simulink model "chan_550_clean"  (Simulink 7.3)
settings: Solver=ode45  StartTime=0.0  StopTime=10000  RelTol=1e-3
undefined symbols (not defined in the file): pfb_coeff_gen_calc
libraries: xbsIndex_r4 x3811, casper_library_misc x151, xps_library x50, casper_library_delays x46, casper_library_flow_control x33, casper_library_reorder x4, casper_library_ffts_twiddle x2
(part 2 of 40)

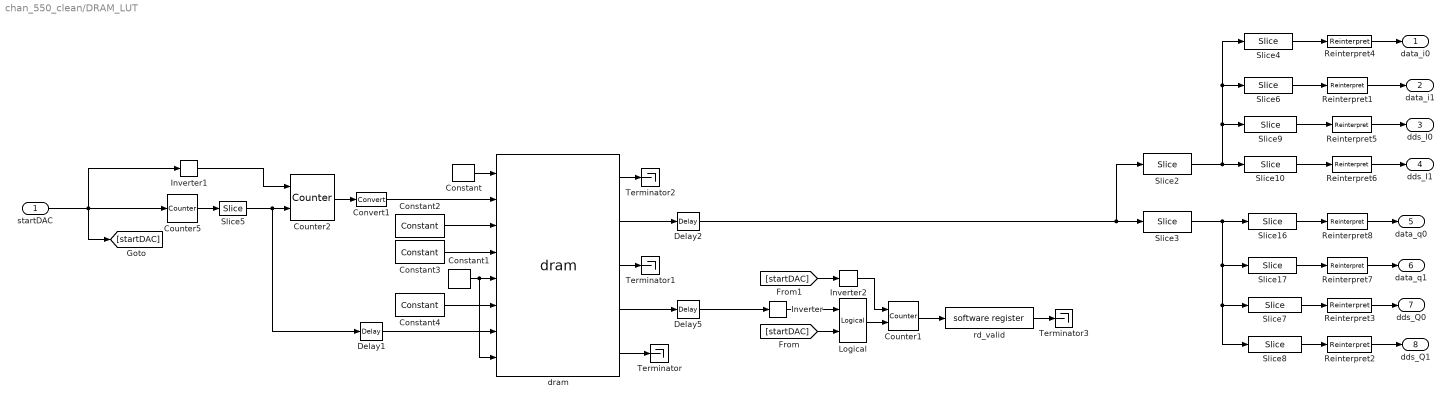
== system chan_550_clean/DRAM_LUT ==
  startDAC : Inport  OutDataType=sfix(16)  OutScaling=2^0
  Constant : xbsIndex_r4/Constant  arith_type=Boolean  const=0  n_bits=16  bin_pt=14  explicit_period=on  period=1  equ=P=C  opselect=C  inp2=PCIN>>17  opr=+  inp1=P  carry=CIN  dbl_ovrd=off  block_type=constant
  Constant1 : xbsIndex_r4/Constant  arith_type=Boolean  const=1  n_bits=16  bin_pt=14  explicit_period=on  period=1  equ=P=C  opselect=C  inp2=PCIN>>17  opr=+  inp1=P  carry=CIN  dbl_ovrd=off  block_type=constant
  Constant2 : xbsIndex_r4/Constant  arith_type=Unsigned  const=0  n_bits=144  bin_pt=0  explicit_period=on  period=1  equ=P=C  opselect=C  inp2=PCIN>>17  opr=+  inp1=P  carry=CIN  dbl_ovrd=off  block_type=constant
  Constant3 : xbsIndex_r4/Constant  arith_type=Unsigned  const=0  n_bits=36  bin_pt=0  explicit_period=on  period=1  equ=P=C  opselect=C  inp2=PCIN>>17  opr=+  inp1=P  carry=CIN  dbl_ovrd=off  block_type=constant
  Constant4 : xbsIndex_r4/Constant  arith_type=Unsigned  const=0  n_bits=32  bin_pt=0  explicit_period=on  period=1  equ=P=C  opselect=C  inp2=PCIN>>17  opr=+  inp1=P  carry=CIN  dbl_ovrd=off  block_type=constant
  Convert1 : xbsIndex_r4/Convert  arith_type=Unsigned  n_bits=32  bin_pt=0  quantization=Truncate  overflow=Wrap  latency=0  dbl_ovrd=off  pipeline=off  block_type=convert
      settings: block_version=11.4
  Counter1 : xbsIndex_r4/Counter  cnt_type=Free Running  cnt_to=511  operation=Up  start_count=0  cnt_by_val=1  arith_type=Unsigned  n_bits=14  bin_pt=0  load_pin=off  rst=on  en=on  explicit_period=on  period=1  dbl_ovrd=off  use_behavioral_HDL=off  implementation=Fabric  use_rpm=off  block_type=counter
  Counter2 : xbsIndex_r4/Counter  cnt_type=Free Running  cnt_to=512  operation=Up  start_count=0  cnt_by_val=1  arith_type=Unsigned  n_bits=14  bin_pt=0  load_pin=off  rst=on  en=on  explicit_period=on  period=1  dbl_ovrd=off  use_behavioral_HDL=off  implementation=Fabric  use_rpm=off  block_type=counter
  Counter5 : xbsIndex_r4/Counter  cnt_type=Free Running  cnt_to=511  operation=Up  start_count=0  cnt_by_val=1  arith_type=Unsigned  n_bits=1  bin_pt=0  load_pin=off  rst=off  en=on  explicit_period=on  period=1  dbl_ovrd=off  use_behavioral_HDL=off  implementation=Fabric  use_rpm=off  block_type=counter
  Delay1 : xbsIndex_r4/Delay  en=off  latency=1  dbl_ovrd=off  reg_retiming=off  block_type=delay
  Delay2 : xbsIndex_r4/Delay  en=off  latency=2  dbl_ovrd=off  reg_retiming=off  block_type=delay
  Delay5 : xbsIndex_r4/Delay  en=off  latency=2  dbl_ovrd=off  reg_retiming=off  block_type=delay
  Inverter : xbsIndex_r4/Inverter  en=off  latency=0  dbl_ovrd=off  block_type=inv
      settings: block_version=11.4
  Inverter1 : xbsIndex_r4/Inverter  en=off  latency=0  dbl_ovrd=off  block_type=inv
      settings: block_version=11.4
  Inverter2 : xbsIndex_r4/Inverter  en=off  latency=0  dbl_ovrd=off  block_type=inv
      settings: block_version=11.4
  Logical : xbsIndex_r4/Logical  logical_function=AND  inputs=2  en=off  latency=0  precision=Full  arith_type=Unsigned  n_bits=16  bin_pt=0  align_bp=on  dbl_ovrd=off  block_type=logical
      settings: block_version=11.4
  Reinterpret{1..8} (8 alike) : xbsIndex_r4/Reinterpret  force_arith_type=on  arith_type="Signed  (2's comp)"  force_bin_pt=on  bin_pt=15  block_type=reinterpret
      settings: infoedit="Changes signal type without altering the binary representation.   You can changed the signal between signed and unsigned, and relocate the binary point.<P><P>Hardware notes: In hardware this block costs nothing.<P><P>Example:  Suppose the input is 6 bits wide, signed, with 2 fractional bits, and the output is forced to unsigned with 0 fractional bits.  Then an input of -2.0 (1110.00 in binary 2's complement) becomes an output of 56 (111000 in binary)."  block_version=10.1.3
  Slice10 : xbsIndex_r4/Slice  nbits=16  boolean_output=off  mode=Lower Bit Location + Width  bit1=0  base1=MSB of Input  bit0=48  base0=LSB of Input  dbl_ovrd=off  block_type=slice
      settings: block_version=10.1.3
  Slice16 : xbsIndex_r4/Slice  nbits=16  boolean_output=off  mode=Lower Bit Location + Width  bit1=0  base1=MSB of Input  bit0=0  base0=LSB of Input  dbl_ovrd=off  block_type=slice
      settings: block_version=10.1.3
  Slice17 : xbsIndex_r4/Slice  nbits=16  boolean_output=off  mode=Lower Bit Location + Width  bit1=0  base1=LSB of Input  bit0=16  base0=LSB of Input  dbl_ovrd=off  block_type=slice
      settings: block_version=10.1.3
  Slice2 : xbsIndex_r4/Slice  nbits=64  boolean_output=off  mode=Lower Bit Location + Width  bit1=0  base1=MSB of Input  bit0=0  base0=LSB of Input  dbl_ovrd=off  block_type=slice
      settings: block_version=10.1.3
  Slice3 : xbsIndex_r4/Slice  nbits=64  boolean_output=off  mode=Lower Bit Location + Width  bit1=0  base1=MSB of Input  bit0=72  base0=LSB of Input  dbl_ovrd=off  block_type=slice
      settings: block_version=10.1.3
  Slice4 : xbsIndex_r4/Slice  nbits=16  boolean_output=off  mode=Lower Bit Location + Width  bit1=0  base1=MSB of Input  bit0=0  base0=LSB of Input  dbl_ovrd=off  block_type=slice
      settings: block_version=10.1.3
  Slice5 : xbsIndex_r4/Slice  nbits=1  boolean_output=on  mode=Lower Bit Location + Width  bit1=0  base1=MSB of Input  bit0=0  base0=LSB of Input  dbl_ovrd=off  block_type=slice
      settings: block_version=10.1.3
  Slice6 : xbsIndex_r4/Slice  nbits=16  boolean_output=off  mode=Lower Bit Location + Width  bit1=0  base1=LSB of Input  bit0=16  base0=LSB of Input  dbl_ovrd=off  block_type=slice
      settings: block_version=10.1.3
  Slice7 : xbsIndex_r4/Slice  nbits=16  boolean_output=off  mode=Lower Bit Location + Width  bit1=0  base1=MSB of Input  bit0=32  base0=LSB of Input  dbl_ovrd=off  block_type=slice
      settings: block_version=10.1.3
  Slice8 : xbsIndex_r4/Slice  nbits=16  boolean_output=off  mode=Lower Bit Location + Width  bit1=0  base1=MSB of Input  bit0=48  base0=LSB of Input  dbl_ovrd=off  block_type=slice
      settings: block_version=10.1.3
  Slice9 : xbsIndex_r4/Slice  nbits=16  boolean_output=off  mode=Lower Bit Location + Width  bit1=0  base1=MSB of Input  bit0=32  base0=LSB of Input  dbl_ovrd=off  block_type=slice
      settings: block_version=10.1.3
  Terminator{,1..3} (4 alike) : Terminator
  dram : xps_library/dram  Tag=xps:dram  SystemSampleTime=-1  dimm=1  arith_type=Unsigned  bin_pt=0  ip_clock=300  sample_period=1  bram_fifos=off  disable_tag=off  use_sim=off  ram_depth=18  bank_mgt=off  wide_data=off  half_burst=off  use_sniffer=on
      link override convert_data_in: arith_type=Unsigned  n_bits=144  bin_pt=0
      link override convert_wr_be: n_bits=18
      link override ddc256_4x_nofir_snap_DRAM_LUT_dram_Mem_Rd_Dout: n_bits=144
      link override ddc256_4x_snap2_DRAM_LUT_dram_Mem_Rd_Dout: n_bits=144
      link override ddc256_4x_snap_fir_DRAM_LUT_dram_Mem_Rd_Dout: n_bits=144
      link override force_rd_dout: arith_type=Unsigned  bin_pt=0
      link override sim_wrapper/dram_sim: sim_method=Inactive  engine_block=ModelSim
  rd_valid : xps_library/software register  Tag=xps:sw_reg  SystemSampleTime=-1  io_dir=To Processor  arith_type=Unsigned  bitwidth=32  bin_pt=0  sample_period=1  gw_name=chan_550_clean_DRAM_LUT_rd_valid_user_data_in
      link override DAC_Final_monitor2_user_data_out: arith_type="Signed  (2's comp)"  n_bits=32  bin_pt=0  period=1
      link override DAC_Final_sync_i_user_data_out: arith_type="Signed  (2's comp)"  n_bits=32  bin_pt=0  period=1
      link override Single_Sine_wave_sync_i_user_data_out: arith_type="Signed  (2's comp)"  n_bits=32  bin_pt=0  period=1
      link override adcToDac2_startDAC_user_data_out: arith_type=Unsigned  n_bits=32  bin_pt=0  period=1
      link override adcToDac4_startDAC_user_data_out: arith_type=Unsigned  n_bits=32  bin_pt=0  period=1
      link override adcToDac5_startDAC_user_data_out: arith_type=Unsigned  n_bits=32  bin_pt=0  period=1
      link override adcToDac6_startDAC_user_data_out: arith_type=Unsigned  n_bits=32  bin_pt=0  period=1
      link override adcToDac7_startDAC_user_data_out: arith_type=Unsigned  n_bits=32  bin_pt=0  period=1
      link override adcToDac_startDAC_user_data_out: arith_type=Unsigned  n_bits=32  bin_pt=0  period=1
      link override adcToDac_sync_i_user_data_out: arith_type="Signed  (2's comp)"  n_bits=32  bin_pt=0  period=1
      link override config10_demo_startDAC_user_data_out: arith_type=Unsigned  n_bits=32  bin_pt=0  period=1
      link override convert: arith_type="Signed  (2's comp)"  n_bits=32  bin_pt=0
      link override dac5681_10_1_software_register_user_data_out: arith_type="Signed  (2's comp)"  n_bits=32  bin_pt=0  period=1
      link override dacADCdemo1_startDAC_user_data_out: arith_type=Unsigned  n_bits=32  bin_pt=0  period=1
      link override dacAdcSnap_startDAC_user_data_out: arith_type=Unsigned  n_bits=32  bin_pt=0  period=1
      link override dacToAdcSnapshot_startDAC_user_data_out: arith_type=Unsigned  n_bits=32  bin_pt=0  period=1
      link override dac_bram_new_block_test_v5_monitor2_user_data_out: arith_type="Signed  (2's comp)"  n_bits=32  bin_pt=0  period=1
      link override dac_bram_new_block_test_v6_monitor2_user_data_out: arith_type="Signed  (2's comp)"  n_bits=32  bin_pt=0  period=1
      link override dac_bram_new_block_test_v7_monitor2_user_data_out: arith_type="Signed  (2's comp)"  n_bits=32  bin_pt=0  period=1
      link override dac_bram_new_block_test_v8_monitor2_user_data_out: arith_type="Signed  (2's comp)"  n_bits=32  bin_pt=0  period=1
      link override dac_bram_new_block_test_v9_monitor2_user_data_out: arith_type="Signed  (2's comp)"  n_bits=32  bin_pt=0  period=1
      link override dac_final_200mhz_sync_i_user_data_out: arith_type="Signed  (2's comp)"  n_bits=32  bin_pt=0  period=1
      link override dac_final_2_sync_i_user_data_out: arith_type="Signed  (2's comp)"  n_bits=32  bin_pt=0  period=1
      link override dac_final_550MHz_V2_sync_i_user_data_out: arith_type="Signed  (2's comp)"  n_bits=32  bin_pt=0  period=1
      link override dac_final_550MHz_sync_i_user_data_out: arith_type="Signed  (2's comp)"  n_bits=32  bin_pt=0  period=1
      link override dac_final_550mhz_delay_sync_i_user_data_out: arith_type="Signed  (2's comp)"  n_bits=32  bin_pt=0  period=1
      link override dac_final_550mhz_new2_sync_i_user_data_out: arith_type="Signed  (2's comp)"  n_bits=32  bin_pt=0  period=1
      link override dac_final_550mhz_new_sync_i_user_data_out: arith_type="Signed  (2's comp)"  n_bits=32  bin_pt=0  period=1
      link override dac_final_550mhz_sync_i_user_data_out: arith_type="Signed  (2's comp)"  n_bits=32  bin_pt=0  period=1
      link override dac_final_sync_i_user_data_out: arith_type="Signed  (2's comp)"  n_bits=32  bin_pt=0  period=1
      link override dac_new_copy2_sync_i_user_data_out: arith_type="Signed  (2's comp)"  n_bits=32  bin_pt=0  period=1
      link override dac_new_copy3_sync_i_user_data_out: arith_type="Signed  (2's comp)"  n_bits=32  bin_pt=0  period=1
      link override dac_new_copy4_sync_i_user_data_out: arith_type="Signed  (2's comp)"  n_bits=32  bin_pt=0  period=1
      link override dac_new_copy_sync_i_user_data_out: arith_type="Signed  (2's comp)"  n_bits=32  bin_pt=0  period=1
      link override dac_new_sync_i_user_data_out: arith_type="Signed  (2's comp)"  n_bits=32  bin_pt=0  period=1
      link override ddc256_4x_b_startDAC_user_data_out: arith_type=Unsigned  n_bits=32  bin_pt=0  period=1
      link override ddc256_4x_c_startDAC_user_data_out: arith_type=Unsigned  n_bits=32  bin_pt=0  period=1
      link override ddc256_4x_nofir_snap_startDAC_user_data_out: arith_type=Unsigned  n_bits=32  bin_pt=0  period=1
      link override ddc256_4x_nofir_startDAC_user_data_out: arith_type=Unsigned  n_bits=32  bin_pt=0  period=1
      link override ddc256_4x_snap2_startDAC_user_data_out: arith_type=Unsigned  n_bits=32  bin_pt=0  period=1
      link override ddc256_4x_snap_fir_startDAC_user_data_out: arith_type=Unsigned  n_bits=32  bin_pt=0  period=1
      link override ddc256_low_sim_startDAC_user_data_out: arith_type=Unsigned  n_bits=32  bin_pt=0  period=1
      link override ddc256_low_startDAC_user_data_out: arith_type=Unsigned  n_bits=32  bin_pt=0  period=1
      link override ddc256_nofir_sim_startDAC_user_data_out: arith_type=Unsigned  n_bits=32  bin_pt=0  period=1
      link override ddc2_startDAC_user_data_out: arith_type=Unsigned  n_bits=32  bin_pt=0  period=1
      link override ddcFull_startDAC_user_data_out: arith_type=Unsigned  n_bits=32  bin_pt=0  period=1
      link override ddcQDR_noise_startDAC_user_data_out: arith_type=Unsigned  n_bits=32  bin_pt=0  period=1
      link override ddc_startDAC_user_data_out: arith_type=Unsigned  n_bits=32  bin_pt=0  period=1
      link override fff_11_complex_startDAC_user_data_out: arith_type=Unsigned  n_bits=32  bin_pt=0  period=1
      link override fff_11_withoutfir_startDAC_user_data_out: arith_type=Unsigned  n_bits=32  bin_pt=0  period=1
      link override fff_32bit_11_withoutfir_new_fft_startDAC_user_data_out: arith_type=Unsigned  n_bits=32  bin_pt=0  period=1
      link override fff_32bit_11_withoutfir_startDAC_user_data_out: arith_type=Unsigned  n_bits=32  bin_pt=0  period=1
      link override fff_test_1_startDAC_user_data_out: arith_type=Unsigned  n_bits=32  bin_pt=0  period=1
      link override fff_working_11_fft_vacc_const4_startDAC_user_data_out: arith_type=Unsigned  n_bits=32  bin_pt=0  period=1
      link override fff_working_11_fft_vacc_long_2_startDAC_user_data_out: arith_type=Unsigned  n_bits=32  bin_pt=0  period=1
      link override fff_working_11_fft_vacc_long_startDAC_user_data_out: arith_type=Unsigned  n_bits=32  bin_pt=0  period=1
      link override fff_working_11_fft_vacc_startDAC_user_data_out: arith_type=Unsigned  n_bits=32  bin_pt=0  period=1
      link override fff_working_11_final_startDAC_user_data_out: arith_type=Unsigned  n_bits=32  bin_pt=0  period=1
      link override ffff_11_startDAC_user_data_out: arith_type=Unsigned  n_bits=32  bin_pt=0  period=1
      link override fftStandard_startDAC_user_data_out: arith_type=Unsigned  n_bits=32  bin_pt=0  period=1
      link override mkid_dac_test_input_data_i_user_data_out: arith_type="Signed  (2's comp)"  n_bits=32  bin_pt=0  period=1
      link override mkid_dac_test_with_ROM_input_data_i_user_data_out: arith_type="Signed  (2's comp)"  n_bits=32  bin_pt=0  period=1
      link override mkid_dac_test_with_ROM_v2_input_data_i_user_data_out: arith_type="Signed  (2's comp)"  n_bits=32  bin_pt=0  period=1
      link override mkid_dac_test_with_ROM_v3_input_data_i_user_data_out: arith_type="Signed  (2's comp)"  n_bits=32  bin_pt=0  period=1
      link override mkid_dac_test_with_ROM_v5_input_data_i_user_data_out: arith_type="Signed  (2's comp)"  n_bits=32  bin_pt=0  period=1
      link override mkid_dac_test_with_ROM_v6_input_data_i_user_data_out: arith_type="Signed  (2's comp)"  n_bits=32  bin_pt=0  period=1
      link override mkid_fft_noloss_11_startDAC_user_data_out: arith_type=Unsigned  n_bits=32  bin_pt=0  period=1
      link override mkid_fft_simulation_startDAC_user_data_out: arith_type=Unsigned  n_bits=32  bin_pt=0  period=1
      link override single_Sine_wave_copy2_100mhz_sync_i_user_data_out: arith_type="Signed  (2's comp)"  n_bits=32  bin_pt=0  period=1
      link override single_Sine_wave_copy2_sync_i_user_data_out: arith_type="Signed  (2's comp)"  n_bits=32  bin_pt=0  period=1
      link override single_Sine_wave_sync_i_user_data_out: arith_type="Signed  (2's comp)"  n_bits=32  bin_pt=0  period=1
  data_i0 : Outport
  data_i1 : Outport  Port=2
  dds_I0 : Outport  Port=3
  dds_I1 : Outport  Port=4
  data_q0 : Outport  Port=5
  data_q1 : Outport  Port=6
  dds_Q0 : Outport  Port=7
  dds_Q1 : Outport  Port=8
  wires:
  Constant/1 -> dram/1
  Constant1/1 -> dram/8, dram/5
  Constant2/1 -> dram/3
  Constant3/1 -> dram/4
  Slice5/1 -> Counter2/2, Delay1/1
  dram/4 -> Delay5/1
  Constant4/1 -> dram/6
  Slice4/1 -> Reinterpret4/1
  Slice6/1 -> Reinterpret1/1
  Slice9/1 -> Reinterpret5/1
  Slice10/1 -> Reinterpret6/1
  Reinterpret4/1 -> data_i0/1
  Reinterpret1/1 -> data_i1/1
  Reinterpret5/1 -> dds_I0/1
  Reinterpret6/1 -> dds_I1/1
  Reinterpret8/1 -> data_q0/1
  Reinterpret7/1 -> data_q1/1
  Reinterpret3/1 -> dds_Q0/1
  Reinterpret2/1 -> dds_Q1/1
  startDAC/1 -> Counter5/1, [startDAC], Inverter1/1
  Counter5/1 -> Slice5/1
  Inverter/1 -> Logical/1
  [startDAC] -> Logical/2
  Convert1/1 -> dram/2
  Slice16/1 -> Reinterpret8/1
  Slice17/1 -> Reinterpret7/1
  Slice7/1 -> Reinterpret3/1
  Slice8/1 -> Reinterpret2/1
  Slice2/1 -> Slice10/1, Slice9/1, Slice6/1, Slice4/1
  Slice3/1 -> Slice16/1, Slice17/1, Slice7/1, Slice8/1
  Counter1/1 -> rd_valid/1
  Logical/1 -> Counter1/2
  [startDAC] -> Inverter2/1
  Inverter2/1 -> Counter1/1
  dram/2 -> Delay2/1
  Counter2/1 -> Convert1/1
  Inverter1/1 -> Counter2/1
  dram/5 -> Terminator/1
  dram/3 -> Terminator1/1
  dram/1 -> Terminator2/1
  rd_valid/1 -> Terminator3/1
  Delay2/1 -> Slice3/1, Slice2/1
  Delay5/1 -> Inverter/1
  Delay1/1 -> dram/7

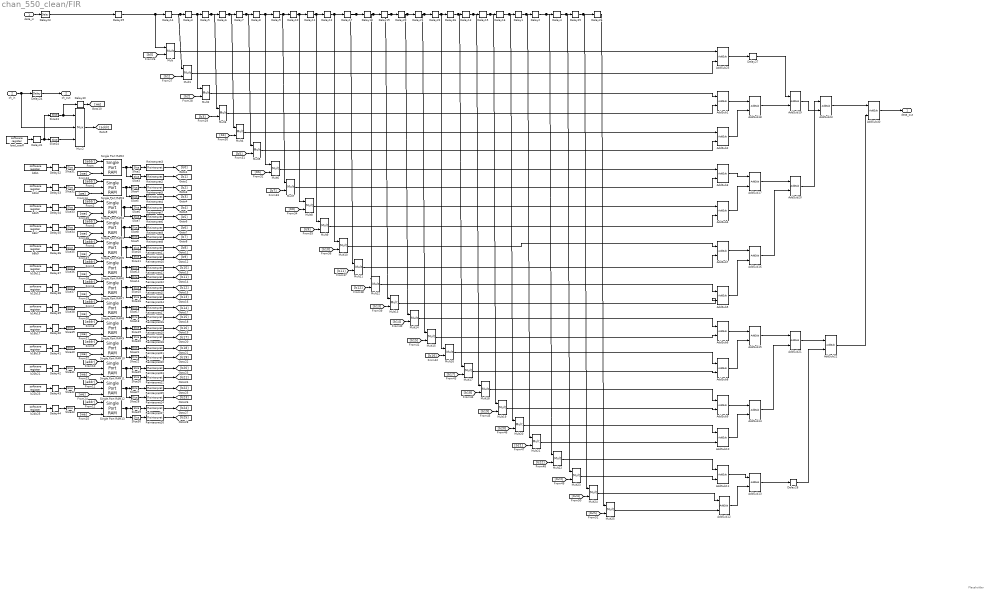
== system chan_550_clean/FIR ==
  note: Placeholder
  data_in : Inport
  ch_in : Inport  Port=2
  AddSub{1..23,25} (24 alike) : xbsIndex_r4/AddSub  mode=Addition  use_carryin=off  use_carryout=off  en=off  latency=3  precision=Full  arith_type="Signed  (2's comp)"  n_bits=16  bin_pt=15  quantization=Truncate  overflow=Wrap  dbl_ovrd=off  use_behavioral_HDL=off  hw_selection=DSP48  pipelined=on  block_type=addsub
  AddSub40 : xbsIndex_r4/AddSub  mode=Addition  use_carryin=off  use_carryout=off  en=off  latency=3  precision=User Defined  arith_type="Signed  (2's comp)"  n_bits=12  bin_pt=9  quantization=Truncate  overflow=Wrap  dbl_ovrd=off  use_behavioral_HDL=off  hw_selection=DSP48  pipelined=on  block_type=addsub
  Delay{1..22,25..26,46} (25 alike) : xbsIndex_r4/Delay  en=off  latency=256  dbl_ovrd=off  reg_retiming=off  block_type=delay
      settings: block_version=11.4
  Delay{23,30..44} (16 alike) : xbsIndex_r4/Delay  en=off  latency=1  dbl_ovrd=off  reg_retiming=off  block_type=delay
      settings: block_version=11.4
  Delay24 : xbsIndex_r4/Delay  en=off  latency=24  dbl_ovrd=off  reg_retiming=off  block_type=delay
      settings: block_version=11.4
  Delay27 : xbsIndex_r4/Delay  en=off  latency=3  dbl_ovrd=off  reg_retiming=off  block_type=delay
      settings: block_version=11.4
  Delay28 : xbsIndex_r4/Delay  en=off  latency=3  dbl_ovrd=off  reg_retiming=off  block_type=delay
      settings: block_version=11.4
  Delay29 : xbsIndex_r4/Delay  en=off  latency=3  dbl_ovrd=off  reg_retiming=off  block_type=delay
      settings: block_version=11.4
  Mult{,1..25} (26 alike) : xbsIndex_r4/Mult  precision=Full  arith_type="Signed  (2's comp)"  n_bits=16  bin_pt=14  quantization=Truncate  overflow=Wrap  en=off  latency=5  dbl_ovrd=off  use_behavioral_HDL=off  opt=Speed  use_embedded=on  optimum_pipeline=on  pipeline=on  use_rpm=on  placement_style=Triangular  block_type=mult
      settings: block_version=11.4
  Mux2 : xbsIndex_r4/Mux  inputs=2  en=off  latency=1  precision=Full  arith_type=Unsigned  n_bits=16  bin_pt=14  quantization=Truncate  overflow=Wrap  dbl_ovrd=off  block_type=mux
      settings: block_version=11.4
  Reinterpret{2..27} (26 alike) : xbsIndex_r4/Reinterpret  force_arith_type=on  arith_type="Signed  (2's comp)"  force_bin_pt=on  bin_pt=11  block_type=reinterpret
      settings: infoedit="Changes signal type without altering the binary representation.   You can changed the signal between signed and unsigned, and relocate the binary point.<br><br>Hardware notes: In hardware this block costs nothing.<br><br>Example:  Suppose the input is 6 bits wide, signed, with 2 fractional bits, and the output is forced to unsigned with 0 fractional bits.  Then an input of -2.0 (1110.00 in binary 2's complement) becomes an output of 56 (111000 in binary)."
  Single Port RAM1 : xbsIndex_r4/Single Port RAM  depth=256  initVector=[97453145 97453145 97453145 97453145 97453145 97453145 97453145 97453145 97453145 97453145]  distributed_mem=Block RAM  write_mode=No Read On Write  rst=off  init_reg=0  en=off  latency=3  dbl_ovrd=off  optimize=Speed  use_rpm=on  block_type=spram
  Single Port RAM10 : xbsIndex_r4/Single Port RAM  depth=256  initVector=[72943055 72943055 72943055 72943055 72943055 72943055 72943055 72943055 72943055 72943055 ]  distributed_mem=Block RAM  write_mode=No Read On Write  rst=off  init_reg=0  en=off  latency=3  dbl_ovrd=off  optimize=Speed  use_rpm=on  block_type=spram
  Single Port RAM11 : xbsIndex_r4/Single Port RAM  depth=256  initVector=[31523587 31523587 31523587 31523587 31523587 31523587 31523587 31523587 31523587 31523587 ]  distributed_mem=Block RAM  write_mode=No Read On Write  rst=off  init_reg=0  en=off  latency=3  dbl_ovrd=off  optimize=Speed  use_rpm=on  block_type=spram
  Single Port RAM12 : xbsIndex_r4/Single Port RAM  depth=256  initVector=[6947073 6947073 6947073 6947073 6947073 6947073 6947073 6947073 6947073 6947073 ]  distributed_mem=Block RAM  write_mode=No Read On Write  rst=off  init_reg=0  en=off  latency=3  dbl_ovrd=off  optimize=Speed  use_rpm=on  block_type=spram
  Single Port RAM13 : xbsIndex_r4/Single Port RAM  depth=256  initVector=[24 24 24 24 24 24 24 24 24 24 ]  distributed_mem=Block RAM  write_mode=No Read On Write  rst=off  init_reg=0  en=off  latency=3  dbl_ovrd=off  optimize=Speed  use_rpm=on  block_type=spram
  Single Port RAM2 : xbsIndex_r4/Single Port RAM  depth=256  initVector=[1572864 1572864 1572864 1572864 1572864 1572864 1572864 1572864 1572864 1572864]  distributed_mem=Block RAM  write_mode=No Read On Write  rst=off  init_reg=0  en=off  latency=3  dbl_ovrd=off  optimize=Speed  use_rpm=on  block_type=spram
  Single Port RAM3 : xbsIndex_r4/Single Port RAM  depth=256  initVector=[16842858 16842858 16842858 16842858 16842858 16842858 16842858 16842858 16842858 16842858]  distributed_mem=Block RAM  write_mode=No Read On Write  rst=off  init_reg=0  en=off  latency=3  dbl_ovrd=off  optimize=Speed  use_rpm=on  block_type=spram
  Single Port RAM4 : xbsIndex_r4/Single Port RAM  depth=256  initVector=[50528737 50528737 50528737 50528737 50528737 50528737 50528737 50528737 50528737 50528737]  distributed_mem=Block RAM  write_mode=No Read On Write  rst=off  init_reg=0  en=off  latency=3  dbl_ovrd=off  optimize=Speed  use_rpm=on  block_type=spram
  Single Port RAM5 : xbsIndex_r4/Single Port RAM  depth=256  initVector=[145098568 145098568 145098568 145098568 145098568 145098568 145098568 145098568 145098568 145098568]  distributed_mem=Block RAM  write_mode=No Read On Write  rst=off  init_reg=0  en=off  latency=3  dbl_ovrd=off  optimize=Speed  use_rpm=on  block_type=spram
  Single Port RAM6 : xbsIndex_r4/Single Port RAM  depth=256  initVector=[178325964 178325964 178325964 178325964 178325964 178325964 178325964 178325964 178325964 178325964]  distributed_mem=Block RAM  write_mode=No Read On Write  rst=off  init_reg=0  en=off  latency=3  dbl_ovrd=off  optimize=Speed  use_rpm=on  block_type=spram
  Single Port RAM7 : xbsIndex_r4/Single Port RAM  depth=256  initVector=[185600784 185600784 185600784 185600784 185600784 185600784 185600784 185600784 185600784 185600784]  distributed_mem=Block RAM  write_mode=No Read On Write  rst=off  init_reg=0  en=off  latency=3  dbl_ovrd=off  optimize=Speed  use_rpm=on  block_type=spram
  Single Port RAM8 : xbsIndex_r4/Single Port RAM  depth=256  initVector=[164367009 164367009 164367009 164367009 164367009 164367009 164367009 164367009 164367009 164367009 ]  distributed_mem=Block RAM  write_mode=No Read On Write  rst=off  init_reg=0  en=off  latency=3  dbl_ovrd=off  optimize=Speed  use_rpm=on  block_type=spram
  Single Port RAM9 : xbsIndex_r4/Single Port RAM  depth=256  initVector=[122161318 122161318 122161318 122161318 122161318 122161318 122161318 122161318 122161318 122161318 ]  distributed_mem=Block RAM  write_mode=No Read On Write  rst=off  init_reg=0  en=off  latency=3  dbl_ovrd=off  optimize=Speed  use_rpm=on  block_type=spram
  Slice{2,4,6,8,10,13,15,17,19,21,25,27,29} (13 alike) : xbsIndex_r4/Slice  nbits=12  boolean_output=off  mode=Lower Bit Location + Width  bit1=0  base1=MSB of Input  bit0=0  base0=LSB of Input  dbl_ovrd=off  block_type=slice
      settings: block_version=10.1.3
  Slice{3,5,7,9,11,14,16,18,20,22,26,28,30} (13 alike) : xbsIndex_r4/Slice  nbits=12  boolean_output=off  mode=Lower Bit Location + Width  bit1=0  base1=MSB of Input  bit0=12  base0=LSB of Input  dbl_ovrd=off  block_type=slice
      settings: block_version=10.1.3
  Slice23 : xbsIndex_r4/Slice  nbits=16  boolean_output=on  mode=Lower Bit Location + Width  bit1=0  base1=MSB of Input  bit0=0  base0=LSB of Input  dbl_ovrd=off  block_type=slice
      settings: block_version=10.1.3
  Slice24 : xbsIndex_r4/Slice  nbits=8  boolean_output=off  mode=Lower Bit Location + Width  bit1=0  base1=MSB of Input  bit0=1  base0=LSB of Input  dbl_ovrd=off  block_type=slice
      settings: block_version=10.1.3
  Slice{31..43} (13 alike) : xbsIndex_r4/Slice  nbits=24  boolean_output=off  mode=Lower Bit Location + Width  bit1=0  base1=MSB of Input  bit0=0  base0=LSB of Input  dbl_ovrd=off  block_type=slice
      settings: block_version=10.1.3
  b0b1 : xps_library/software register  Tag=xps:sw_reg  SystemSampleTime=-1  io_dir=From Processor  arith_type=Unsigned  bitwidth=32  bin_pt=0  sample_period=1  gw_name=chan_550_clean_FIR_b0b1_user_data_out
      link override bruno_mod5_dest_port_user_data_out: arith_type=Unsigned  n_bits=32  bin_pt=0  period=1
      link override dumpTo10Gbe_dest_port_user_data_out: arith_type=Unsigned  n_bits=32  bin_pt=0  period=1
      link override r_10gbe_demo_dest_port_user_data_out: arith_type=Unsigned  n_bits=32  bin_pt=0  period=1
      link override r_10gbe_test_dest_port_user_data_out: arith_type=Unsigned  n_bits=32  bin_pt=0  period=1
      link override r_xaui_test_cnt_rst_user_data_out: arith_type=Unsigned  n_bits=32  bin_pt=0  period=1
      link override tut3_dest_port_user_data_out: arith_type=Unsigned  n_bits=32  bin_pt=0  period=1
  b10b11 : xps_library/software register  Tag=xps:sw_reg  SystemSampleTime=-1  io_dir=From Processor  arith_type=Unsigned  bitwidth=32  bin_pt=0  sample_period=1  gw_name=chan_550_clean_FIR_b10b11_user_data_out
      link override bruno_mod5_dest_port_user_data_out: arith_type=Unsigned  n_bits=32  bin_pt=0  period=1
      link override dumpTo10Gbe_dest_port_user_data_out: arith_type=Unsigned  n_bits=32  bin_pt=0  period=1
      link override r_10gbe_demo_dest_port_user_data_out: arith_type=Unsigned  n_bits=32  bin_pt=0  period=1
      link override r_10gbe_test_dest_port_user_data_out: arith_type=Unsigned  n_bits=32  bin_pt=0  period=1
      link override r_xaui_test_cnt_rst_user_data_out: arith_type=Unsigned  n_bits=32  bin_pt=0  period=1
      link override tut3_dest_port_user_data_out: arith_type=Unsigned  n_bits=32  bin_pt=0  period=1
  b12b13 : xps_library/software register  Tag=xps:sw_reg  SystemSampleTime=-1  io_dir=From Processor  arith_type=Unsigned  bitwidth=32  bin_pt=0  sample_period=1  gw_name=chan_550_clean_FIR_b12b13_user_data_out
      link override bruno_mod5_dest_port_user_data_out: arith_type=Unsigned  n_bits=32  bin_pt=0  period=1
      link override dumpTo10Gbe_dest_port_user_data_out: arith_type=Unsigned  n_bits=32  bin_pt=0  period=1
      link override r_10gbe_demo_dest_port_user_data_out: arith_type=Unsigned  n_bits=32  bin_pt=0  period=1
      link override r_10gbe_test_dest_port_user_data_out: arith_type=Unsigned  n_bits=32  bin_pt=0  period=1
      link override r_xaui_test_cnt_rst_user_data_out: arith_type=Unsigned  n_bits=32  bin_pt=0  period=1
      link override tut3_dest_port_user_data_out: arith_type=Unsigned  n_bits=32  bin_pt=0  period=1
  b14b15 : xps_library/software register  Tag=xps:sw_reg  SystemSampleTime=-1  io_dir=From Processor  arith_type=Unsigned  bitwidth=32  bin_pt=0  sample_period=1  gw_name=chan_550_clean_FIR_b14b15_user_data_out
      link override bruno_mod5_dest_port_user_data_out: arith_type=Unsigned  n_bits=32  bin_pt=0  period=1
      link override dumpTo10Gbe_dest_port_user_data_out: arith_type=Unsigned  n_bits=32  bin_pt=0  period=1
      link override r_10gbe_demo_dest_port_user_data_out: arith_type=Unsigned  n_bits=32  bin_pt=0  period=1
      link override r_10gbe_test_dest_port_user_data_out: arith_type=Unsigned  n_bits=32  bin_pt=0  period=1
      link override r_xaui_test_cnt_rst_user_data_out: arith_type=Unsigned  n_bits=32  bin_pt=0  period=1
      link override tut3_dest_port_user_data_out: arith_type=Unsigned  n_bits=32  bin_pt=0  period=1
  b16b17 : xps_library/software register  Tag=xps:sw_reg  SystemSampleTime=-1  io_dir=From Processor  arith_type=Unsigned  bitwidth=32  bin_pt=0  sample_period=1  gw_name=chan_550_clean_FIR_b16b17_user_data_out
      link override bruno_mod5_dest_port_user_data_out: arith_type=Unsigned  n_bits=32  bin_pt=0  period=1
      link override dumpTo10Gbe_dest_port_user_data_out: arith_type=Unsigned  n_bits=32  bin_pt=0  period=1
      link override r_10gbe_demo_dest_port_user_data_out: arith_type=Unsigned  n_bits=32  bin_pt=0  period=1
      link override r_10gbe_test_dest_port_user_data_out: arith_type=Unsigned  n_bits=32  bin_pt=0  period=1
      link override r_xaui_test_cnt_rst_user_data_out: arith_type=Unsigned  n_bits=32  bin_pt=0  period=1
      link override tut3_dest_port_user_data_out: arith_type=Unsigned  n_bits=32  bin_pt=0  period=1
  b18b19 : xps_library/software register  Tag=xps:sw_reg  SystemSampleTime=-1  io_dir=From Processor  arith_type=Unsigned  bitwidth=32  bin_pt=0  sample_period=1  gw_name=chan_550_clean_FIR_b18b19_user_data_out
      link override bruno_mod5_dest_port_user_data_out: arith_type=Unsigned  n_bits=32  bin_pt=0  period=1
      link override dumpTo10Gbe_dest_port_user_data_out: arith_type=Unsigned  n_bits=32  bin_pt=0  period=1
      link override r_10gbe_demo_dest_port_user_data_out: arith_type=Unsigned  n_bits=32  bin_pt=0  period=1
      link override r_10gbe_test_dest_port_user_data_out: arith_type=Unsigned  n_bits=32  bin_pt=0  period=1
      link override r_xaui_test_cnt_rst_user_data_out: arith_type=Unsigned  n_bits=32  bin_pt=0  period=1
      link override tut3_dest_port_user_data_out: arith_type=Unsigned  n_bits=32  bin_pt=0  period=1
  b20b21 : xps_library/software register  Tag=xps:sw_reg  SystemSampleTime=-1  io_dir=From Processor  arith_type=Unsigned  bitwidth=32  bin_pt=0  sample_period=1  gw_name=chan_550_clean_FIR_b20b21_user_data_out
      link override bruno_mod5_dest_port_user_data_out: arith_type=Unsigned  n_bits=32  bin_pt=0  period=1
      link override dumpTo10Gbe_dest_port_user_data_out: arith_type=Unsigned  n_bits=32  bin_pt=0  period=1
      link override r_10gbe_demo_dest_port_user_data_out: arith_type=Unsigned  n_bits=32  bin_pt=0  period=1
      link override r_10gbe_test_dest_port_user_data_out: arith_type=Unsigned  n_bits=32  bin_pt=0  period=1
      link override r_xaui_test_cnt_rst_user_data_out: arith_type=Unsigned  n_bits=32  bin_pt=0  period=1
      link override tut3_dest_port_user_data_out: arith_type=Unsigned  n_bits=32  bin_pt=0  period=1
  b22b23 : xps_library/software register  Tag=xps:sw_reg  SystemSampleTime=-1  io_dir=From Processor  arith_type=Unsigned  bitwidth=32  bin_pt=0  sample_period=1  gw_name=chan_550_clean_FIR_b22b23_user_data_out
      link override bruno_mod5_dest_port_user_data_out: arith_type=Unsigned  n_bits=32  bin_pt=0  period=1
      link override dumpTo10Gbe_dest_port_user_data_out: arith_type=Unsigned  n_bits=32  bin_pt=0  period=1
      link override r_10gbe_demo_dest_port_user_data_out: arith_type=Unsigned  n_bits=32  bin_pt=0  period=1
      link override r_10gbe_test_dest_port_user_data_out: arith_type=Unsigned  n_bits=32  bin_pt=0  period=1
      link override r_xaui_test_cnt_rst_user_data_out: arith_type=Unsigned  n_bits=32  bin_pt=0  period=1
      link override tut3_dest_port_user_data_out: arith_type=Unsigned  n_bits=32  bin_pt=0  period=1
  b24b25 : xps_library/software register  Tag=xps:sw_reg  SystemSampleTime=-1  io_dir=From Processor  arith_type=Unsigned  bitwidth=32  bin_pt=0  sample_period=1  gw_name=chan_550_clean_FIR_b24b25_user_data_out
      link override bruno_mod5_dest_port_user_data_out: arith_type=Unsigned  n_bits=32  bin_pt=0  period=1
      link override dumpTo10Gbe_dest_port_user_data_out: arith_type=Unsigned  n_bits=32  bin_pt=0  period=1
      link override r_10gbe_demo_dest_port_user_data_out: arith_type=Unsigned  n_bits=32  bin_pt=0  period=1
      link override r_10gbe_test_dest_port_user_data_out: arith_type=Unsigned  n_bits=32  bin_pt=0  period=1
      link override r_xaui_test_cnt_rst_user_data_out: arith_type=Unsigned  n_bits=32  bin_pt=0  period=1
      link override tut3_dest_port_user_data_out: arith_type=Unsigned  n_bits=32  bin_pt=0  period=1
  b2b3 : xps_library/software register  Tag=xps:sw_reg  SystemSampleTime=-1  io_dir=From Processor  arith_type=Unsigned  bitwidth=32  bin_pt=0  sample_period=1  gw_name=chan_550_clean_FIR_b2b3_user_data_out
      link override bruno_mod5_dest_port_user_data_out: arith_type=Unsigned  n_bits=32  bin_pt=0  period=1
      link override dumpTo10Gbe_dest_port_user_data_out: arith_type=Unsigned  n_bits=32  bin_pt=0  period=1
      link override r_10gbe_demo_dest_port_user_data_out: arith_type=Unsigned  n_bits=32  bin_pt=0  period=1
      link override r_10gbe_test_dest_port_user_data_out: arith_type=Unsigned  n_bits=32  bin_pt=0  period=1
      link override r_xaui_test_cnt_rst_user_data_out: arith_type=Unsigned  n_bits=32  bin_pt=0  period=1
      link override tut3_dest_port_user_data_out: arith_type=Unsigned  n_bits=32  bin_pt=0  period=1
  b4b5 : xps_library/software register  Tag=xps:sw_reg  SystemSampleTime=-1  io_dir=From Processor  arith_type=Unsigned  bitwidth=32  bin_pt=0  sample_period=1  gw_name=chan_550_clean_FIR_b4b5_user_data_out
      link override bruno_mod5_dest_port_user_data_out: arith_type=Unsigned  n_bits=32  bin_pt=0  period=1
      link override dumpTo10Gbe_dest_port_user_data_out: arith_type=Unsigned  n_bits=32  bin_pt=0  period=1
      link override r_10gbe_demo_dest_port_user_data_out: arith_type=Unsigned  n_bits=32  bin_pt=0  period=1
      link override r_10gbe_test_dest_port_user_data_out: arith_type=Unsigned  n_bits=32  bin_pt=0  period=1
      link override r_xaui_test_cnt_rst_user_data_out: arith_type=Unsigned  n_bits=32  bin_pt=0  period=1
      link override tut3_dest_port_user_data_out: arith_type=Unsigned  n_bits=32  bin_pt=0  period=1
  b6b7 : xps_library/software register  Tag=xps:sw_reg  SystemSampleTime=-1  io_dir=From Processor  arith_type=Unsigned  bitwidth=32  bin_pt=0  sample_period=1  gw_name=chan_550_clean_FIR_b6b7_user_data_out
      link override bruno_mod5_dest_port_user_data_out: arith_type=Unsigned  n_bits=32  bin_pt=0  period=1
      link override dumpTo10Gbe_dest_port_user_data_out: arith_type=Unsigned  n_bits=32  bin_pt=0  period=1
      link override r_10gbe_demo_dest_port_user_data_out: arith_type=Unsigned  n_bits=32  bin_pt=0  period=1
      link override r_10gbe_test_dest_port_user_data_out: arith_type=Unsigned  n_bits=32  bin_pt=0  period=1
      link override r_xaui_test_cnt_rst_user_data_out: arith_type=Unsigned  n_bits=32  bin_pt=0  period=1
      link override tut3_dest_port_user_data_out: arith_type=Unsigned  n_bits=32  bin_pt=0  period=1
  b8b9 : xps_library/software register  Tag=xps:sw_reg  SystemSampleTime=-1  io_dir=From Processor  arith_type=Unsigned  bitwidth=32  bin_pt=0  sample_period=1  gw_name=chan_550_clean_FIR_b8b9_user_data_out
      link override bruno_mod5_dest_port_user_data_out: arith_type=Unsigned  n_bits=32  bin_pt=0  period=1
      link override dumpTo10Gbe_dest_port_user_data_out: arith_type=Unsigned  n_bits=32  bin_pt=0  period=1
      link override r_10gbe_demo_dest_port_user_data_out: arith_type=Unsigned  n_bits=32  bin_pt=0  period=1
      link override r_10gbe_test_dest_port_user_data_out: arith_type=Unsigned  n_bits=32  bin_pt=0  period=1
      link override r_xaui_test_cnt_rst_user_data_out: arith_type=Unsigned  n_bits=32  bin_pt=0  period=1
      link override tut3_dest_port_user_data_out: arith_type=Unsigned  n_bits=32  bin_pt=0  period=1
  load_coeff : xps_library/software register  Tag=xps:sw_reg  SystemSampleTime=-1  io_dir=From Processor  arith_type=Unsigned  bitwidth=32  bin_pt=0  sample_period=1  gw_name=chan_550_clean_FIR_load_coeff_user_data_out
      link override bruno_mod5_dest_port_user_data_out: arith_type=Unsigned  n_bits=32  bin_pt=0  period=1
      link override dumpTo10Gbe_dest_port_user_data_out: arith_type=Unsigned  n_bits=32  bin_pt=0  period=1
      link override r_10gbe_demo_dest_port_user_data_out: arith_type=Unsigned  n_bits=32  bin_pt=0  period=1
      link override r_10gbe_test_dest_port_user_data_out: arith_type=Unsigned  n_bits=32  bin_pt=0  period=1
      link override r_xaui_test_cnt_rst_user_data_out: arith_type=Unsigned  n_bits=32  bin_pt=0  period=1
      link override tut3_dest_port_user_data_out: arith_type=Unsigned  n_bits=32  bin_pt=0  period=1
  data_out : Outport
  ch_out : Outport  Port=2
  wires:
  data_in/1 -> Delay23/1
  ch_in/1 -> Mux2/2, Delay24/1
  Delay24/1 -> ch_out/1
  load_coeff/1 -> Delay31/1
  Slice23/1 -> Mux2/1, Delay30/1
  Slice24/1 -> Mux2/3
  b0b1/1 -> Delay32/1
  b2b3/1 -> Delay33/1
  b4b5/1 -> Delay34/1
  b6b7/1 -> Delay35/1
  b8b9/1 -> Delay36/1
  b10b11/1 -> Delay37/1
  b12b13/1 -> Delay38/1
  b14b15/1 -> Delay39/1
  b16b17/1 -> Delay40/1
  b18b19/1 -> Delay41/1
  b20b21/1 -> Delay42/1
  b22b23/1 -> Delay43/1
  b24b25/1 -> Delay44/1
  Single Port RAM2/1 -> Slice2/1, Slice3/1
  Slice2/1 -> Reinterpret3/1
  Slice3/1 -> Reinterpret2/1
  Single Port RAM3/1 -> Slice4/1, Slice5/1
  Slice4/1 -> Reinterpret5/1
  Slice5/1 -> Reinterpret4/1
  Single Port RAM4/1 -> Slice6/1, Slice7/1
  Slice6/1 -> Reinterpret7/1
  Slice7/1 -> Reinterpret6/1
  Single Port RAM1/1 -> Slice9/1, Slice8/1
  Slice8/1 -> Reinterpret9/1
  Slice9/1 -> Reinterpret8/1
  Single Port RAM5/1 -> Slice10/1, Slice11/1
  Slice10/1 -> Reinterpret11/1
  Slice11/1 -> Reinterpret10/1
  Single Port RAM6/1 -> Slice14/1, Slice13/1
  Slice13/1 -> Reinterpret13/1
  Slice14/1 -> Reinterpret12/1
  Single Port RAM7/1 -> Slice16/1, Slice15/1
  Slice15/1 -> Reinterpret15/1
  Slice16/1 -> Reinterpret14/1
  Single Port RAM8/1 -> Slice17/1, Slice18/1
  Slice17/1 -> Reinterpret17/1
  Slice18/1 -> Reinterpret16/1
  Single Port RAM9/1 -> Slice19/1, Slice20/1
  Slice19/1 -> Reinterpret19/1
  Slice20/1 -> Reinterpret18/1
  Single Port RAM10/1 -> Slice22/1, Slice21/1
  Slice21/1 -> Reinterpret21/1
  Slice22/1 -> Reinterpret20/1
  Single Port RAM11/1 -> Slice26/1, Slice25/1
  Slice25/1 -> Reinterpret23/1
  Slice26/1 -> Reinterpret22/1
  Single Port RAM12/1 -> Slice27/1, Slice28/1
  Slice27/1 -> Reinterpret25/1
  Slice28/1 -> Reinterpret24/1
  Single Port RAM13/1 -> Slice30/1, Slice29/1
  Slice29/1 -> Reinterpret27/1
  Slice30/1 -> Reinterpret26/1
  Mux2/1 -> [addr]
  [addr] -> Single Port RAM2/1
  [addr] -> Single Port RAM3/1
  [addr] -> Single Port RAM4/1
  [addr] -> Single Port RAM1/1
  [addr] -> Single Port RAM5/1
  [addr] -> Single Port RAM6/1
  [addr] -> Single Port RAM7/1
  [addr] -> Single Port RAM9/1
  [addr] -> Single Port RAM8/1
  [addr] -> Single Port RAM10/1
  [addr] -> Single Port RAM11/1
  [addr] -> Single Port RAM12/1
  [addr] -> Single Port RAM13/1
  [we] -> Single Port RAM2/3
  [we] -> Single Port RAM3/3
  [we] -> Single Port RAM4/3
  [we] -> Single Port RAM1/3
  [we] -> Single Port RAM5/3
  [we] -> Single Port RAM6/3
  [we] -> Single Port RAM7/3
  [we] -> Single Port RAM8/3
  [we] -> Single Port RAM9/3
  [we] -> Single Port RAM10/3
  [we] -> Single Port RAM11/3
  [we] -> Single Port RAM12/3
  [we] -> Single Port RAM13/3
  Delay14/1 -> Mult1/1, Delay3/1
  Delay23/1 -> Delay29/1
  [b4] -> Mult4/2
  [b5] -> Mult5/2
  [b6] -> Mult6/2
  [b7] -> Mult7/2
  [b8] -> Mult8/2
  [b9] -> Mult9/2
  [b10] -> Mult10/2
  [b11] -> Mult11/2
  [b12] -> Mult12/2
  [b13] -> Mult13/2
  [b14] -> Mult14/2
  [b15] -> Mult15/2
  [b16] -> Mult16/2
  [b17] -> Mult17/2
  [b18] -> Mult18/2
  [b19] -> Mult19/2
  [b20] -> Mult20/2
  [b21] -> Mult21/2
  [b22] -> Mult22/2
  [b23] -> Mult23/2
  [b24] -> Mult24/2
  [b25] -> Mult25/2
  Mult24/1 -> AddSub12/1
  Reinterpret3/1 -> [b0]
  Reinterpret2/1 -> [b1]
  Reinterpret5/1 -> [b2]
  Reinterpret4/1 -> [b3]
  Reinterpret7/1 -> [b4]
  Reinterpret6/1 -> [b5]
  Reinterpret9/1 -> [b6]
  Reinterpret8/1 -> [b7]
  Reinterpret11/1 -> [b8]
  Reinterpret10/1 -> [b9]
  Reinterpret13/1 -> [b10]
  Reinterpret12/1 -> [b11]
  Reinterpret15/1 -> [b12]
  Reinterpret14/1 -> [b13]
  Reinterpret17/1 -> [b14]
  Reinterpret16/1 -> [b15]
  Reinterpret19/1 -> [b16]
  Reinterpret18/1 -> [b17]
  Reinterpret21/1 -> [b18]
  Reinterpret20/1 -> [b19]
  Reinterpret23/1 -> [b20]
  Reinterpret22/1 -> [b21]
  Reinterpret25/1 -> [b22]
  Reinterpret24/1 -> [b23]
  Reinterpret27/1 -> [b24]
  Reinterpret26/1 -> [b25]
  [b0] -> Mult/2
  [b1] -> Mult1/2
  [b2] -> Mult2/2
  [b3] -> Mult3/2
  Delay30/1 -> [we]
  Delay31/1 -> Slice23/1, Slice24/1
  Delay32/1 -> Slice31/1
  Delay33/1 -> Slice32/1
  Delay34/1 -> Slice33/1
  Delay35/1 -> Slice34/1
  Delay36/1 -> Slice35/1
  Delay37/1 -> Slice36/1
  Delay38/1 -> Slice37/1
  Delay39/1 -> Slice38/1
  Delay40/1 -> Slice39/1
  Delay41/1 -> Slice40/1
  Delay42/1 -> Slice41/1
  Delay43/1 -> Slice42/1
  Delay44/1 -> Slice43/1
  Delay29/1 -> Delay14/1, Mult/1
  Slice31/1 -> Single Port RAM2/2
  Slice32/1 -> Single Port RAM3/2
  Slice33/1 -> Single Port RAM4/2
  Slice34/1 -> Single Port RAM1/2
  Slice35/1 -> Single Port RAM5/2
  Slice36/1 -> Single Port RAM6/2
  Slice37/1 -> Single Port RAM7/2
  Slice38/1 -> Single Port RAM8/2
  Slice39/1 -> Single Port RAM9/2
  Slice40/1 -> Single Port RAM10/2
  Slice41/1 -> Single Port RAM11/2
  Slice42/1 -> Single Port RAM12/2
  Slice43/1 -> Single Port RAM13/2
  ? -> ?
  Delay3/1 -> Mult2/1, Delay5/1
  Delay5/1 -> Mult3/1, Delay6/1
  Delay6/1 -> Mult4/1, Delay7/1
  Delay7/1 -> Mult5/1, Delay8/1
  Delay8/1 -> Delay9/1, Mult6/1
  Delay9/1 -> Delay10/1, Mult7/1
  Delay10/1 -> Delay11/1, Mult8/1
  Delay11/1 -> Delay12/1, Mult9/1
  Delay12/1 -> Mult10/1, Delay17/1
  Delay17/1 -> Delay18/1, Mult11/1
  Delay18/1 -> Delay19/1, Mult12/1
  Delay19/1 -> Delay20/1, Mult13/1
  Delay20/1 -> Delay21/1, Mult14/1
  Delay21/1 -> Delay22/1, Mult15/1
  Delay22/1 -> Delay46/1, Mult16/1
  Delay46/1 -> Delay13/1, Mult17/1
  Delay13/1 -> Delay15/1, Mult18/1
  Delay15/1 -> Delay16/1, Mult19/1
  Delay26/1 -> Mult25/1
  Delay16/1 -> Delay1/1, Mult20/1
  Delay1/1 -> Delay2/1, Mult21/1
  Delay2/1 -> Delay4/1, Mult22/1
  Delay4/1 -> Delay25/1, Mult23/1
  Delay25/1 -> Delay26/1, Mult24/1
  Mult/1 -> AddSub25/1
  Mult1/1 -> AddSub25/2
  Mult2/1 -> AddSub1/1
  Mult3/1 -> AddSub1/2
  Mult4/1 -> AddSub2/1
  Mult5/1 -> AddSub2/2
  Mult6/1 -> AddSub3/1
  Mult7/1 -> AddSub3/2
  Mult8/1 -> AddSub4/1
  Mult9/1 -> AddSub4/2
  Mult10/1 -> AddSub7/1
  Mult11/1 -> AddSub7/2
  Mult12/1 -> AddSub5/1
  Mult13/1 -> AddSub5/2
  Mult14/1 -> AddSub6/1
  Mult15/1 -> AddSub6/2
  Mult16/1 -> AddSub8/1
  Mult17/1 -> AddSub8/2
  Mult18/1 -> AddSub9/1
  Mult19/1 -> AddSub9/2
  Mult20/1 -> AddSub10/1
  Mult21/1 -> AddSub10/2
  Mult22/1 -> AddSub11/1
  Mult23/1 -> AddSub11/2
  Mult25/1 -> AddSub12/2
  AddSub11/1 -> AddSub13/1
  AddSub12/1 -> AddSub13/2
  AddSub9/1 -> AddSub14/1
  AddSub10/1 -> AddSub14/2
  AddSub6/1 -> AddSub15/1
  AddSub8/1 -> AddSub15/2
  AddSub7/1 -> AddSub16/1
  AddSub5/1 -> AddSub16/2
  AddSub3/1 -> AddSub17/1
  AddSub4/1 -> AddSub17/2
  AddSub1/1 -> AddSub18/1
  AddSub2/1 -> AddSub18/2
  AddSub25/1 -> Delay27/1
  Delay27/1 -> AddSub19/1
  AddSub18/1 -> AddSub19/2
  AddSub17/1 -> AddSub20/1
  AddSub16/1 -> AddSub20/2
  AddSub15/1 -> AddSub21/1
  AddSub14/1 -> AddSub21/2
  AddSub21/1 -> AddSub22/1
  AddSub13/1 -> Delay28/1
  Delay28/1 -> AddSub22/2
  AddSub19/1 -> AddSub23/2
  AddSub20/1 -> AddSub23/1
  AddSub22/1 -> AddSub40/2
  AddSub23/1 -> AddSub40/1
  AddSub40/1 -> data_out/1
